simulink library "ee_lib_for_power_systems"  (R2025b)
settings: Solver=VariableStepAuto  StartTime=0.0  StopTime=10.0  RelTol=1e-3
undefined symbols (not defined in the file or in its folder): Alt_PolePair_Count, C3m, DiscIntNeverNeededParam, Enum1to12, L3m, Total_SM_Inertia, Ts
libraries: fl_lib x291, nesl_utility x219, ee_lib x139, custom_lib x12, $bdroot x10, ee_sl_lib x3, eeTransforms x2, eeGeneralControl x1, simulink x1
(part 5 of 11)

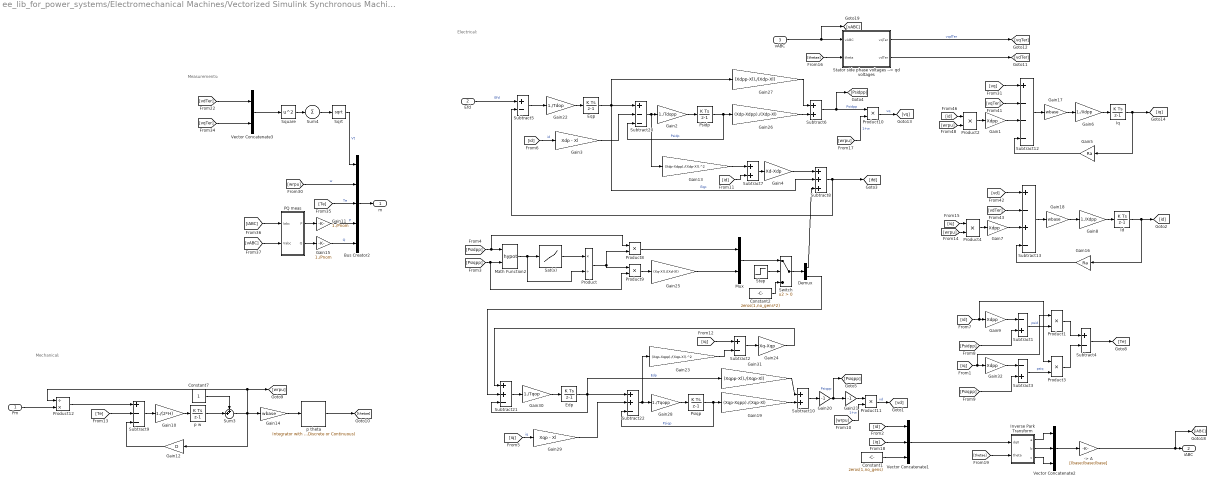
== system ee_lib_for_power_systems/Electromechanical Machines/Vectorized Simulink Synchronous Machine (PU) ==
  note: Mechanical:
  note: Electrical:
  note: Measurements:
  Pm : Inport
  Efd : Inport  Port=2
  vABC : Inport  Port=3
  -> A : Gain  Gain=[Ibase;Ibase;Ibase]
  Bus Creator2 : BusCreator  Inputs=5
  Constant1 : Constant  Value=zeros(1,no_gens)  VectorParams1D=off  SampleTime=Ts
  Constant2 : Constant  Value=zeros(1,no_gens*2)  VectorParams1D=off  SampleTime=Ts
  Constant7 : Constant  VectorParams1D=off  SampleTime=Ts
  Demux : Demux  Outputs=2
  Edp : DiscreteIntegrator  SampleTime=Ts  ICPrevOutput=DiscIntNeverNeededParam  ICPrevScaledInput=DiscIntNeverNeededParam
  Eqp : DiscreteIntegrator  SampleTime=Ts  ICPrevOutput=DiscIntNeverNeededParam  ICPrevScaledInput=DiscIntNeverNeededParam
  Gain{1,7,9,32} (4 alike) : Gain  Gain=Xdpp
  Gain10 : Gain  Gain=1./(2*H)
  Gain11 : Gain  Gain=1./Pnom
  Gain12 : Gain  Gain=D
  Gain13 : Gain  Gain=(Xdp-Xdpp)./(Xdp-Xl).^2
  Gain14 : Gain  Gain=wbase
  Gain15 : Gain  Gain=1./Pnom
  Gain16 : Gain  Gain=Ra
  Gain17 : Gain  Gain=wbase
  Gain18 : Gain  Gain=wbase
  Gain19 : Gain  Gain=(Xqp-Xqpp)./(Xqp-Xl)
  Gain2 : Gain  Gain=1./Tdopp
  Gain20 : Gain  Gain=-1
  Gain21 : Gain  Gain=-1
  Gain22 : Gain  Gain=1./Tdop
  Gain23 : Gain  Gain=(Xqp-Xqpp)./(Xqp-Xl).^2
  Gain24 : Gain  Gain=Xq-Xqp
  Gain25 : Gain  Gain=(Xq-Xl)./(Xd-Xl)
  Gain26 : Gain  Gain=(Xdp-Xdpp)./(Xdp-Xl)
  Gain27 : Gain  Gain=(Xdpp-Xl)./(Xdp-Xl)
  Gain28 : Gain  Gain=1./Tqopp
  Gain29 : Gain  Gain=Xqp - Xl
  Gain3 : Gain  Gain=Xdp - Xl
  Gain30 : Gain  Gain=1./Tqop
  Gain31 : Gain  Gain=(Xqpp-Xl)./(Xqp-Xl)
  Gain4 : Gain  Gain=Xd-Xdp
  Gain5 : Gain  Gain=Ra
  Gain6 : Gain  Gain=1./Xdpp
  Gain8 : Gain  Gain=1./Xdpp
  Id : DiscreteIntegrator  SampleTime=Ts  ICPrevOutput=DiscIntNeverNeededParam  ICPrevScaledInput=DiscIntNeverNeededParam
  Inverse Park Transform : Subsystem  mask{no_meas=no_gens}
      mask prompts: no_meas "Number of Measurements"
      settings: LibrarySourceBlock=|
      | ee_sl_lib/Mathematical
      | Transforms/Inverse
      | Park Transform
  Iq : DiscreteIntegrator  SampleTime=Ts  ICPrevOutput=DiscIntNeverNeededParam  ICPrevScaledInput=DiscIntNeverNeededParam
  Math Function2 : Math  Operator=hypot  SignedPower=on
  Mux : Mux  Inputs=2
  PQ meas : Subsystem  DialogController=POWERSYS.PowerSysDialog
      settings: LibrarySourceBlock=|
      | sps_lib/Sensors and Measurements/Power
      | (3ph, Instantaneous)
  Product : Product  Inputs=*/
  Product1 : Product
  Product{2,4,8..11} (6 alike) : Product  RndMeth=Zero
  Product12 : Product  Inputs=/*
  Product3 : Product
  Psidp : DiscreteIntegrator  SampleTime=Ts  ICPrevOutput=DiscIntNeverNeededParam  ICPrevScaledInput=DiscIntNeverNeededParam
  Psiqp : DiscreteIntegrator  SampleTime=Ts  ICPrevOutput=DiscIntNeverNeededParam  ICPrevScaledInput=DiscIntNeverNeededParam
  Sat(x) : Lookup_n-D  NumberOfTableDimensions=1  LookupTableObject=''  BreakpointsForDimension1=xVec  Table=SatX  InternalRulePriority=Speed  RndMeth=Simplest
  Sqrt : Sqrt
  Square : Math  Operator=square
  Stator side phase voltages --> qd voltages : Subsystem
  Step : Step  SampleTime=Ts
  Subtract1 : Sum  Inputs=-+
  Subtract10 : Sum
  Subtract12 : Sum  Inputs=+---
  Subtract13 : Sum  Inputs=+-+-
  Subtract2 : Sum  Inputs=+-
  Subtract20 : Sum  Inputs=+--
  Subtract21 : Sum  Inputs=++-
  Subtract22 : Sum  Inputs=++-
  Subtract3 : Sum  Inputs=-+
  Subtract4 : Sum  Inputs=+-
  Subtract5 : Sum  Inputs=+-
  Subtract6 : Sum
  Subtract7 : Sum
  Subtract8 : Sum  Inputs=+++
  Subtract9 : Sum  Inputs=+--
  Sum3 : Sum  Inputs=++|
  Sum4 : Sum  Inputs=1  CollapseMode=Specified dimension  InputSameDT=on  SaturateOnIntegerOverflow=on  OutDataTypeStr=Inherit: Same as first input
  Switch : Switch  Criteria=u2 > Threshold  SaturateOnIntegerOverflow=off  InputSameDT=off
  Vector Concatenate1 : Concatenate  NumInputs=3  Mode=Multidimensional array
  Vector Concatenate2 : Concatenate  NumInputs=3  Mode=Multidimensional array
  Vector Concatenate3 : Concatenate  Mode=Multidimensional array
  "p theta " : eeGeneralControl/Integrator with Wrapped State (Discrete or Continuous)  LabelModeActiveChoice=Discrete  WrappedStateUpperValue=2*pi  WrappedStateLowerValue=0  x0=0  Ts=Ts
      settings: LibrarySourceBlock=|
      | eeSinusoidalMeasurementV2/Sinusoidal Measurement
      | (PLL)/Angle/Integrator with
      | Wrapped State
      | (Discrete or Continuous)
  p w : DiscreteIntegrator  gainval=1  SampleTime=-1  ICPrevOutput=DiscIntNeverNeededParam  ICPrevScaledInput=DiscIntNeverNeededParam
  m : Outport
  iABC : Outport  Port=2
  wires:
  Constant2/1 -> Switch/3
  Demux/2 -> Subtract21/2
  Demux/1 -> Subtract8/3
  Switch/1 -> Demux/1
  Step/1 -> Switch/2
  Mux/1 -> Switch/1
  Gain25/1 -> Mux/2
  Product8/1 -> Mux/1
  Product/1 -> Product9/1, Product8/2
  Math Function2/1 -> Product/2, Sat(x)/1
  Subtract4/1 -> [Te]
  Product1/1 -> Subtract4/1
  Product3/1 -> Subtract4/2
  [Psiqpp] -> Subtract3/2
  Gain32/1 -> Subtract3/1
  [iq] -> Gain32/1, Product1/1
  Subtract3/1 -> Product3/2  "psiq"
  Subtract1/1 -> Product1/2  "psid"
  Sqrt/1 -> Bus Creator2/1  "Vt"
  Sat(x)/1 -> Product/1
  [thetae] -> Inverse Park Transform/2(theta)
  [thetae] -> Stator side phase voltages --> qd voltages/2(theta)
  "p theta "/1 -> [thetae]
  [vdTer] -> Subtract13/2
  Subtract13/1 -> Gain18/1
  Gain24/1 -> Subtract21/1
  Product4/1 -> Gain7/1
  Gain22/1 -> Eqp/1
  [iq] -> Product4/1
  [id] -> Gain9/1, Product3/1
  Psiqp/1 -> Subtract22/3, Gain19/1  "Psiqp"
  Gain28/1 -> Psiqp/1
  [iq] -> Gain29/1  "iq"
  Gain9/1 -> Subtract1/1
  Product9/1 -> Gain25/1
  [id] -> Subtract7/2
  Id/1 -> Gain16/1, [id]
  Gain21/1 -> Product11/1
  Gain1/1 -> Subtract12/3
  Gain2/1 -> Psidp/1
  Iq/1 -> [iq], Gain5/1
  [wrpu] -> Product4/2
  Gain8/1 -> Id/1
  [Psidpp] -> Subtract1/2
  [wrpu] -> Product11/2  "1+w"
  [vq] -> Subtract12/1
  Gain16/1 -> Subtract13/4
  [id] -> Product2/1
  Gain6/1 -> Iq/1
  [vqTer] -> Subtract12/2
  Product2/1 -> Gain1/1
  [wrpu] -> Product2/2
  Gain5/1 -> Subtract12/4
  Subtract5/1 -> Gain22/1
  Gain30/1 -> Edp/1
  Product10/1 -> [vq]  "vq"
  Product11/1 -> [vd]  "vd"
  [wrpu] -> Product10/2  "1+w"
  Gain17/1 -> Gain6/1
  [vd] -> Subtract13/1
  Gain31/1 -> Subtract10/1
  Gain18/1 -> Gain8/1
  Subtract21/1 -> Gain30/1
  Gain4/1 -> Subtract8/1
  Subtract7/1 -> Gain4/1
  Efd/1 -> Subtract5/1  "Efd"
  Gain3/1 -> Subtract20/2
  Eqp/1 -> Gain27/1, Subtract20/1, Subtract8/2  "Eqp"
  Subtract8/1 -> Subtract5/2, [ifd]
  Subtract2/1 -> Gain24/1
  Gain29/1 -> Subtract22/2
  [Psidpp] -> Math Function2/1, Product8/1
  [id] -> Gain3/1  "id"
  [Psiqpp] -> Math Function2/2, Product9/2
  Subtract22/1 -> Gain28/1, Gain23/1
  Subtract6/1 -> Product10/1, [Psidpp]  "Psidpp"
  Subtract20/1 -> Gain2/1, Gain13/1
  Gain19/1 -> Subtract10/2
  Gain13/1 -> Subtract7/1
  Gain26/1 -> Subtract6/2
  Gain7/1 -> Subtract13/3
  Gain27/1 -> Subtract6/1
  Subtract12/1 -> Gain17/1
  Gain20/1 -> Gain21/1, [Psiqpp]  "Psiqpp"
  Subtract10/1 -> Gain20/1
  [iq] -> Subtract2/1
  Psidp/1 -> Gain26/1, Subtract20/3  "Psidp"
  Gain23/1 -> Subtract2/2
  Edp/1 -> Subtract21/3, Subtract22/1, Gain31/1  "Edp"
  [Te] -> Subtract9/2
  Vector Concatenate1/1 -> Inverse Park Transform/1(dq0)
  -> A/1 -> iABC/1, [iABC]
  vABC/1 -> Stator side phase voltages --> qd voltages/1(vABC), [vABC]
  Stator side phase voltages --> qd voltages/2(vdTer) -> [vdTer]
  Gain15/1 -> Bus Creator2/5  "Q"
  Gain11/1 -> Bus Creator2/4  "P"
  PQ meas/1(Q) -> Gain15/1
  PQ meas/2(P) -> Gain11/1
  [vABC] -> PQ meas/1(Vabc)
  [iABC] -> PQ meas/2(Iabc)
  [Te] -> Bus Creator2/3  "Te"
  [wrpu] -> Bus Creator2/2  "w"
  [vqTer] -> Vector Concatenate3/2
  Vector Concatenate2/1 -> -> A/1
  Inverse Park Transform/3(c) -> Vector Concatenate2/3
  Inverse Park Transform/1(a) -> Vector Concatenate2/1
  Inverse Park Transform/2(b) -> Vector Concatenate2/2
  Constant7/1 -> Sum3/1
  Stator side phase voltages --> qd voltages/1(vqTer) -> [vqTer]  "vqdTer"
  Sum3/1 -> Gain12/1, Gain14/1, [wrpu], Product12/1
  Pm/1 -> Product12/2
  p w/1 -> Sum3/2
  Subtract9/1 -> Gain10/1
  Gain10/1 -> p w/1
  Product12/1 -> Subtract9/1
  Gain12/1 -> Subtract9/3
  Gain14/1 -> "p theta "/1
  [id] -> Vector Concatenate1/1
  [iq] -> Vector Concatenate1/2
  Bus Creator2/1 -> m/1
  [vdTer] -> Vector Concatenate3/1
  Constant1/1 -> Vector Concatenate1/3
  Vector Concatenate3/1 -> Square/1
  Square/1 -> Sum4/1
  Sum4/1 -> Sqrt/1

== system ee_lib_for_power_systems/Network Components/ Three-Winding Transformer PU (Three-Phase) ==
  Transformer : ee_lib/Passive/Transformers/Three-Winding Transformer (Three-Phase)  SRated=Pnom_Fnom(1) A*V  FRated=Pnom_Fnom(2) Hz  Winding1Connection=ee.enum.windingconnection.Yg  VRated1=V1 V  Winding2Connection=ee.enum.windingconnection.delta1  VRated2=V2 V  Winding3Connection=ee.enum.windingconnection.Y  VRated3=V3 V  CoreType=ee.enum.coretype.fivelimb  pu_Rw1=R1_pu  pu_Rw2=R2_pu  pu_Rw3=R3_pu  leakage_reactance_option=ee.enum.transformer_leakage.include  pu_Xl1=L1_pu  pu_Xl2=L2_pu  pu_Xl3=L3_pu  magnetizing_resistance_option=ee.enum.transformer_magnetizingResistance.include  pu_Rm=Rm_pu  magnetizing_reactance_option=ee.enum.transformer_magnetizingReactance.include  saturation_option=ee.enum.transformer_saturation.exclude  pu_Xm=Lm_pu  current_data=[0, .002, 1, 2]  magnetic_flux_data=[0, 1.2, 1.5, 1.51]  pu_X0=0.5  i1_specify=off  i1_priority=None  i1=[0, 0, 0] A  i1_nominal_specify=off  i1_nominal_value=1  i1_nominal_unit=A  i2_specify=off  i2_priority=None  i2=[0, 0, 0] A  i2_nominal_specify=off  i2_nominal_value=1  i2_nominal_unit=A  i3_specify=off  i3_priority=None  i3=[0, 0, 0] A  i3_nominal_specify=off  i3_nominal_value=1  i3_nominal_unit=A  phi_specify=off  phi_priority=None  phi=[0, 0, 0]  phi_nominal_specify=off  phi_nominal_value=1  phi_nominal_unit=1  power_dissipated_specify=off  power_dissipated_priority=None  power_dissipated=0 W  power_dissipated_nominal_specify=off  power_dissipated_nominal_value=1  power_dissipated_nominal_unit=W
      settings: InternalSimscapePortConfiguration="{\"Bottom\":[],\"Left\":[{\"id\":\"N1\",\"label\":\"~1\",\"type\":\"foundation.electrical.three_phase\"}],\"Right\":[{\"id\":\"N2\",\"label\":\"~2\",\"type\":\"foundation.electrical.three_phase\"},{\"id\":\"N3\",\"label\":\"~3\",\"type\":\"foundation.electrical.three_phase\"}],\"Top\":[]}"
  ~1 : PMIOPort  Side=Left
  ~2 : PMIOPort  Port=2  Side=Right
  ~3 : PMIOPort  Port=3  Side=Right
  connections:
  Transformer/LConn1 -- ~1/RConn1
  ~2/RConn1 -- Transformer/RConn1
  ~3/RConn1 -- Transformer/RConn2

== system ee_lib_for_power_systems/Network Components/3-Phase Branch Positive Sequence PU ==
  C1 : ee_lib/Passive/RLC Assemblies/RLC (Three-Phase)  port_option=ee.enum.threePhasePort.composite  component_structure=ee.enum.rlc.structure.C  R=1 Ohm  L=1e-3 H  C=B/(Vnom2^2/Pnom_Fnom(1))/(2*pi*Pnom_Fnom(2))/2 F  R_parasitic=0 Ohm  G_parasitic=0 1/Ohm  IL_specify=off  IL_priority=High  IL=[0, 0, 0] A  IL_nominal_specify=off  IL_nominal_value=1  IL_nominal_unit=A  VC_specify=on  VC_priority=Low  VC=[0, 0, 0] V  VC_nominal_specify=off  VC_nominal_value=1  VC_nominal_unit=V
      settings: InternalSimscapePortConfiguration="{\"Bottom\":[],\"Left\":[{\"id\":\"N1\",\"label\":\"~1\",\"type\":\"foundation.electrical.three_phase\"}],\"Right\":[{\"id\":\"N2\",\"label\":\"~2\",\"type\":\"foundation.electrical.three_phase\"}],\"Top\":[]}"
  C2 : ee_lib/Passive/RLC Assemblies/RLC (Three-Phase)  port_option=ee.enum.threePhasePort.composite  component_structure=ee.enum.rlc.structure.C  R=1 Ohm  L=1e-3 H  C=B/(Vnom2^2/Pnom_Fnom(1))/(2*pi*Pnom_Fnom(2))/2 F  R_parasitic=0 Ohm  G_parasitic=0 1/Ohm  IL_specify=off  IL_priority=High  IL=[0, 0, 0] A  IL_nominal_specify=off  IL_nominal_value=1  IL_nominal_unit=A  VC_specify=on  VC_priority=Low  VC=[0, 0, 0] V  VC_nominal_specify=off  VC_nominal_value=1  VC_nominal_unit=V
      settings: InternalSimscapePortConfiguration="{\"Bottom\":[],\"Left\":[{\"id\":\"N1\",\"label\":\"~1\",\"type\":\"foundation.electrical.three_phase\"}],\"Right\":[{\"id\":\"N2\",\"label\":\"~2\",\"type\":\"foundation.electrical.three_phase\"}],\"Top\":[]}"
  RLC : ee_lib/Passive/RLC Assemblies/RLC (Three-Phase)  port_option=ee.enum.threePhasePort.composite  component_structure=ee.enum.rlc.structure.SeriesRL  R=R*Vnom2^2/Pnom_Fnom(1) Ohm  L=X*Vnom2^2/Pnom_Fnom(1)/(2*pi*Pnom_Fnom(2)) H  C=-1/(Vnom2^2/Pnom_Fnom(1))/(2*pi*Pnom_Fnom(2))/X F  R_parasitic=0 Ohm  G_parasitic=0 1/Ohm  IL_specify=on  IL_priority=Low  IL=[0, 0, 0] A  IL_nominal_specify=off  IL_nominal_value=1  IL_nominal_unit=A  VC_specify=on  VC_priority=Low  VC=[0, 0, 0] V  VC_nominal_specify=off  VC_nominal_value=1  VC_nominal_unit=V
      settings: InternalSimscapePortConfiguration="{\"Bottom\":[],\"Left\":[{\"id\":\"N1\",\"label\":\"~1\",\"type\":\"foundation.electrical.three_phase\"}],\"Right\":[{\"id\":\"N2\",\"label\":\"~2\",\"type\":\"foundation.electrical.three_phase\"}],\"Top\":[]}"
  Tx : Subsystem  Commented=through  mask{wr=Vnom1/Vnom2}
      mask prompts: wr "Winding Ratio (V1/V2)"
  gnd1 : ee_lib/Connectors & References/Grounded Neutral (Three-Phase)
  gnd2 : ee_lib/Connectors & References/Grounded Neutral (Three-Phase)
  ~1 : PMIOPort  Side=Left
  ~2 : PMIOPort  Port=2  Side=Right
  connections:
  Tx/RConn1 -- RLC/LConn1 -- C1/LConn1
  Tx/LConn1 -- ~1/RConn1
  RLC/RConn1 -- ~2/RConn1 -- C2/LConn1
  gnd1/LConn1 -- C1/RConn1
  gnd2/LConn1 -- C2/RConn1

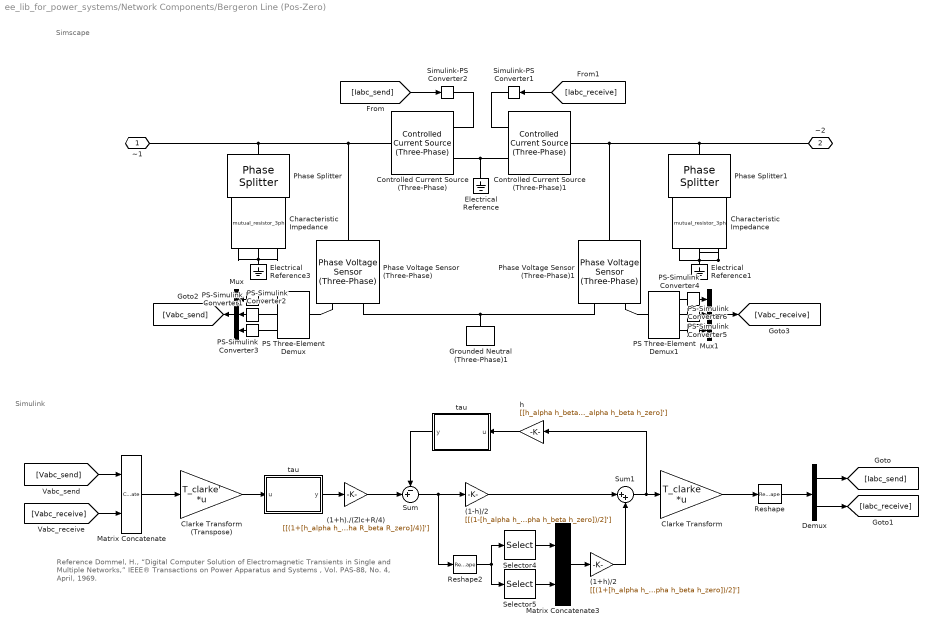
== system ee_lib_for_power_systems/Network Components/Bergeron Line (Pos-Zero) ==
  note: Simulink
  note: Simscape
  note: Reference
      |
      | Dommel, H., “Digital Computer Solution of Electromagnetic Transients in Single and Multiple Networks,” IEEE® Transactions on Power Apparatus and Systems , Vol. PAS-88, No. 4, April, 1969.
  (1+h)./(Zlc+R/4) : Gain  Gain=[[(1+[h_alpha h_beta h_zero])./([Zlc_alpha Zlc_beta Zlc_zero]+[R_alpha R_beta R_zero]/4)]' [(1+[h_alpha h_beta h_zero])./([Zlc_alpha Zlc_beta Zlc_zero]+[R_alpha R_beta R_zero]/4)]']
  (1+h)/2 : Gain  Gain=[[(1+[h_alpha h_beta h_zero])/2]' [(1+[h_alpha h_beta h_zero])/2]']
  (1-h)/2 : Gain  Gain=[[(1-[h_alpha h_beta h_zero])/2]' [(1-[h_alpha h_beta h_zero])/2]']
  Characteristic Impedance : custom_lib/mutual_resistor_3ph  R=Zc Ohm  V_specify=off  V_priority=None  V=zeros(3, 1) V  V_nominal_specify=off  V_nominal_value=1  V_nominal_unit=V  I_specify=off  I_priority=High  I=zeros(3, 1) A  I_nominal_specify=off  I_nominal_value=1  I_nominal_unit=A
      settings: InternalSimscapePortConfiguration="{\"Bottom\":[],\"Left\":[{\"id\":\"p1\",\"label\":\"p1\",\"type\":\"foundation.electrical.electrical\"},{\"id\":\"p2\",\"label\":\"p2\",\"type\":\"foundation.electrical.electrical\"},{\"id\":\"p3\",\"label\":\"p3\",\"type\":\"foundation.electrical.electrical\"}],\"Right\":[{\"id\":\"n1\",\"label\":\"n1\",\"type\":\"foundation.electrical.electrical\"},{\"id\":\"n2\",\"label\":\"n2\",\"type\":\"foundation.electrical.electrical\"},{\"id\":\"n3\",\"label\":\"n3\",\"type\":\"foundation.electrical.electrical\"}],\"Top\":[]}"
  "Characteristic Impedance " : custom_lib/mutual_resistor_3ph  R=Zc Ohm  V_specify=off  V_priority=None  V=zeros(3, 1) V  V_nominal_specify=off  V_nominal_value=1  V_nominal_unit=V  I_specify=off  I_priority=High  I=zeros(3, 1) A  I_nominal_specify=off  I_nominal_value=1  I_nominal_unit=A
      settings: InternalSimscapePortConfiguration="{\"Bottom\":[],\"Left\":[{\"id\":\"p1\",\"label\":\"p1\",\"type\":\"foundation.electrical.electrical\"},{\"id\":\"p2\",\"label\":\"p2\",\"type\":\"foundation.electrical.electrical\"},{\"id\":\"p3\",\"label\":\"p3\",\"type\":\"foundation.electrical.electrical\"}],\"Right\":[{\"id\":\"n1\",\"label\":\"n1\",\"type\":\"foundation.electrical.electrical\"},{\"id\":\"n2\",\"label\":\"n2\",\"type\":\"foundation.electrical.electrical\"},{\"id\":\"n3\",\"label\":\"n3\",\"type\":\"foundation.electrical.electrical\"}],\"Top\":[]}"
  Clarke Transform (Transpose) : Gain  Gain=T_clarke'  Multiplication=Matrix(K*u)
  "Clarke Transform " : Gain  Gain=T_clarke  Multiplication=Matrix(K*u)
  Controlled Current Source (Three-Phase) : ee_lib/Sources/Controlled Current Source (Three-Phase)  input_option=ee.enum.inputType.instantaneous  FRated=60 Hz
      settings: InternalSimscapePortConfiguration="{\"Bottom\":[],\"Left\":[{\"id\":\"S\",\"label\":\"S\",\"type\":\"input\"},{\"id\":\"n\",\"label\":\"n\",\"type\":\"foundation.electrical.electrical\"}],\"Right\":[{\"id\":\"N\",\"label\":\"~\",\"type\":\"foundation.electrical.three_phase\"}],\"Top\":[]}"
  Controlled Current Source (Three-Phase)1 : ee_lib/Sources/Controlled Current Source (Three-Phase)  input_option=ee.enum.inputType.instantaneous  FRated=60 Hz
      settings: InternalSimscapePortConfiguration="{\"Bottom\":[],\"Left\":[{\"id\":\"S\",\"label\":\"S\",\"type\":\"input\"},{\"id\":\"n\",\"label\":\"n\",\"type\":\"foundation.electrical.electrical\"}],\"Right\":[{\"id\":\"N\",\"label\":\"~\",\"type\":\"foundation.electrical.three_phase\"}],\"Top\":[]}"
  Demux : Demux  Outputs=2
  Electrical Reference : fl_lib/Electrical/Electrical Elements/Electrical Reference
  Electrical Reference1 : fl_lib/Electrical/Electrical Elements/Electrical Reference
  Electrical Reference3 : fl_lib/Electrical/Electrical Elements/Electrical Reference
  Grounded Neutral (Three-Phase)1 : ee_lib/Connectors & References/Grounded Neutral (Three-Phase)
  Matrix Concatenate : Concatenate  Mode=Multidimensional array  ConcatenateDimension=2
  Matrix Concatenate3 : Concatenate  Mode=Multidimensional array  ConcatenateDimension=2
  Mux : Mux  Inputs=3
  Mux1 : Mux  Inputs=3
  PS Three-Element Demux : fl_lib/Physical Signals/Nonlinear Operators/PS Three-Element Demux
      settings: InternalSimscapePortConfiguration="{\"Bottom\":[],\"Left\":[{\"id\":\"abc\",\"label\":\"abc\",\"type\":\"input\"}],\"Right\":[{\"id\":\"a\",\"label\":\"a\",\"type\":\"output\"},{\"id\":\"b\",\"label\":\"b\",\"type\":\"output\"},{\"id\":\"c\",\"label\":\"c\",\"type\":\"output\"}],\"Top\":[]}"
  PS Three-Element Demux1 : fl_lib/Physical Signals/Nonlinear Operators/PS Three-Element Demux
      settings: InternalSimscapePortConfiguration="{\"Bottom\":[],\"Left\":[{\"id\":\"abc\",\"label\":\"abc\",\"type\":\"input\"}],\"Right\":[{\"id\":\"a\",\"label\":\"a\",\"type\":\"output\"},{\"id\":\"b\",\"label\":\"b\",\"type\":\"output\"},{\"id\":\"c\",\"label\":\"c\",\"type\":\"output\"}],\"Top\":[]}"
  PS-Simulink Converter{1..6} (6 alike) : nesl_utility/PS-Simulink Converter  PhysicalDomain=network_engine_domain  SubClassName=ps_output  LeftPortType=input  RightPortType=output  PseudoPeriodic=off  Frequencies=[]  VectorFormat=inherit  Unit=inherit  AffineConversion=off
  Phase Splitter : ee_lib/Connectors & References/Phase Splitter
  Phase Splitter1 : ee_lib/Connectors & References/Phase Splitter
  Phase Voltage Sensor (Three-Phase) : ee_lib/Sensors & Transducers/Phase Voltage Sensor (Three-Phase)
  Phase Voltage Sensor (Three-Phase)1 : ee_lib/Sensors & Transducers/Phase Voltage Sensor (Three-Phase)
  Reshape : Reshape
  Reshape2 : Reshape
  Selector4 : Selector  InputPortWidth=6  IndexOptions=Index vector (dialog)  Indices=4:6  OutputSizes=1
  Selector5 : Selector  InputPortWidth=6  IndexOptions=Index vector (dialog)  Indices=1:3  OutputSizes=1
  Simulink-PS Converter1 : nesl_utility/Simulink-PS Converter  PhysicalDomain=network_engine_domain  SubClassName=ps_input  LeftPortType=input  RightPortType=output  PseudoPeriodic=off  Frequencies=[]  NoiseDistribution=none  NoiseParameters=[]  Unit=1  AffineConversion=off  FilteringAndDerivatives=provide  SimscapeFilterOrder=2  InputFilterTimeConstant=0.001  UdotUserProvided=0
  Simulink-PS Converter2 : nesl_utility/Simulink-PS Converter  PhysicalDomain=network_engine_domain  SubClassName=ps_input  LeftPortType=input  RightPortType=output  PseudoPeriodic=off  Frequencies=[]  NoiseDistribution=none  NoiseParameters=[]  Unit=1  AffineConversion=off  FilteringAndDerivatives=provide  SimscapeFilterOrder=2  InputFilterTimeConstant=0.001  UdotUserProvided=0
  Sum : Sum  Inputs=-+|
  Sum1 : Sum  Inputs=|++
  h : Gain  Gain=[[h_alpha h_beta h_zero]' [h_alpha h_beta h_zero]']
  tau : Subsystem  mask{tau=[tau_alpha tau_beta tau_zero], Ts=Ts}
      mask prompts: tau "Transport Delay (s)"; Ts "Sample Time (s)"
      mask DialogControl DescGroupVar Prompt: Discrete Transport Delay
      mask DialogControl DescTextVar Prompt: This block implements a discrete transport delay. When the transport delay is not an integer multiple of the sample time, the remainder is averaged.
      mask DialogControl param Prompt: Parameters
      mask Display: disp('Discrete\nTransport\nDelay')
  "tau " : Subsystem  mask{tau=[tau_alpha tau_beta tau_zero], Ts=Ts}
      mask prompts: tau "Transport Delay (s)"; Ts "Sample Time (s)"
      mask DialogControl DescGroupVar Prompt: Discrete Transport Delay
      mask DialogControl DescTextVar Prompt: This block implements a discrete transport delay. When the transport delay is not an integer multiple of the sample time, the remainder is averaged.
      mask DialogControl param Prompt: Parameters
      mask Display: disp('Discrete\nTransport\nDelay')
  ~1 : PMIOPort  Side=Left
  ~2 : PMIOPort  Port=2  Side=Right
  wires:
  Selector5/1 -> Matrix Concatenate3/2
  Matrix Concatenate/1 -> Clarke Transform (Transpose)/1
  (1-h)/2/1 -> Sum1/1
  (1+h)./(Zlc+R/4)/1 -> Sum/2
  Demux/2 -> [Iabc_receive]
  Demux/1 -> [Iabc_send]
  Selector4/1 -> Matrix Concatenate3/1
  "Clarke Transform "/1 -> Reshape/1
  Reshape/1 -> Demux/1
  "tau "/1(y) -> Sum/1
  [Vabc_send] -> Matrix Concatenate/1
  (1+h)/2/1 -> Sum1/2
  Matrix Concatenate3/1 -> (1+h)/2/1
  h/1 -> "tau "/1(u)
  tau/1(y) -> (1+h)./(Zlc+R/4)/1
  Reshape2/1 -> Selector4/1, Selector5/1
  Sum1/1 -> "Clarke Transform "/1, h/1
  [Vabc_receive] -> Matrix Concatenate/2
  Sum/1 -> (1-h)/2/1, Reshape2/1
  Clarke Transform (Transpose)/1 -> tau/1(u)
  Mux/1 -> [Vabc_send]
  Mux1/1 -> [Vabc_receive]
  PS-Simulink Converter2/1 -> Mux/2
  PS-Simulink Converter1/1 -> Mux/1
  PS-Simulink Converter3/1 -> Mux/3
  PS-Simulink Converter5/1 -> Mux1/2
  PS-Simulink Converter6/1 -> Mux1/3
  PS-Simulink Converter4/1 -> Mux1/1
  [Iabc_send] -> Simulink-PS Converter2/1
  [Iabc_receive] -> Simulink-PS Converter1/1
  connections:
  "Characteristic Impedance "/LConn1 -- Phase Splitter1/RConn1
  "Characteristic Impedance "/LConn2 -- Phase Splitter1/RConn2
  "Characteristic Impedance "/LConn3 -- Phase Splitter1/RConn3
  Electrical Reference1/LConn1 -- "Characteristic Impedance "/RConn2 -- "Characteristic Impedance "/RConn1 -- "Characteristic Impedance "/RConn3
  Characteristic Impedance/LConn3 -- Phase Splitter/RConn3
  Characteristic Impedance/LConn2 -- Phase Splitter/RConn2
  Characteristic Impedance/LConn1 -- Phase Splitter/RConn1
  Characteristic Impedance/RConn2 -- Characteristic Impedance/RConn3 -- Characteristic Impedance/RConn1 -- Electrical Reference3/LConn1
  PS Three-Element Demux1/LConn1 -- Phase Voltage Sensor (Three-Phase)1/RConn1
  ~1/RConn1 -- Phase Splitter/LConn1 -- Phase Voltage Sensor (Three-Phase)/LConn1 -- Controlled Current Source (Three-Phase)/RConn1
  PS-Simulink Converter6/LConn1 -- PS Three-Element Demux1/RConn3
  PS-Simulink Converter3/LConn1 -- PS Three-Element Demux/RConn3
  PS-Simulink Converter2/LConn1 -- PS Three-Element Demux/RConn2
  PS-Simulink Converter5/LConn1 -- PS Three-Element Demux1/RConn2
  PS-Simulink Converter4/LConn1 -- PS Three-Element Demux1/RConn1
  PS Three-Element Demux/LConn1 -- Phase Voltage Sensor (Three-Phase)/RConn1
  Controlled Current Source (Three-Phase)1/RConn1 -- Phase Splitter1/LConn1 -- ~2/RConn1 -- Phase Voltage Sensor (Three-Phase)1/LConn1
  Grounded Neutral (Three-Phase)1/LConn1 -- Phase Voltage Sensor (Three-Phase)/RConn2 -- Phase Voltage Sensor (Three-Phase)1/RConn2
  PS-Simulink Converter1/LConn1 -- PS Three-Element Demux/RConn1
  Simulink-PS Converter2/RConn1 -- Controlled Current Source (Three-Phase)/LConn1
  Controlled Current Source (Three-Phase)/LConn2 -- Controlled Current Source (Three-Phase)1/LConn2 -- Electrical Reference/LConn1
  Controlled Current Source (Three-Phase)1/LConn1 -- Simulink-PS Converter1/RConn1

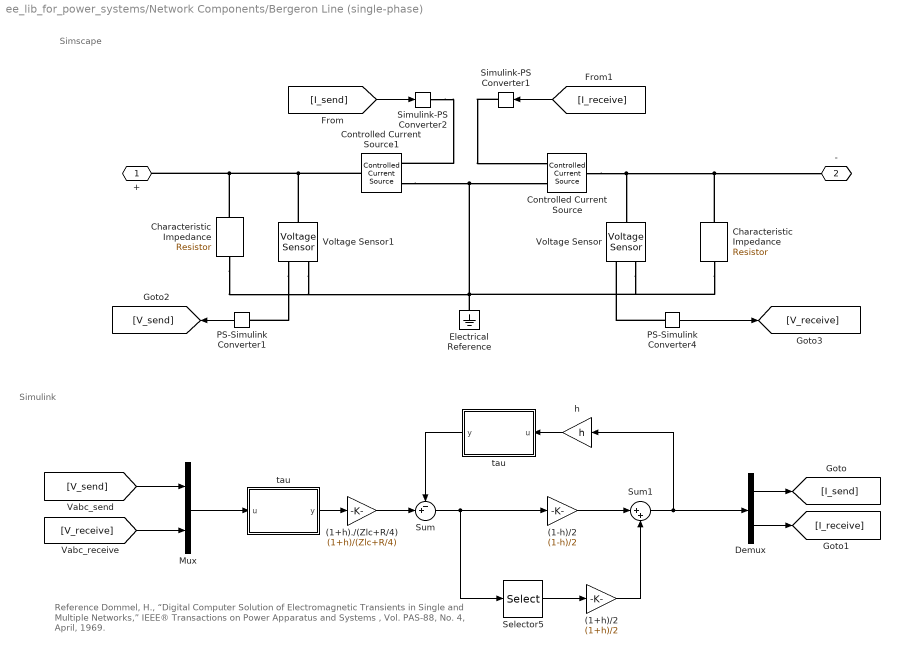
== system ee_lib_for_power_systems/Network Components/Bergeron Line (single-phase) ==
  note: Reference
      |
      | Dommel, H., “Digital Computer Solution of Electromagnetic Transients in Single and Multiple Networks,” IEEE® Transactions on Power Apparatus and Systems , Vol. PAS-88, No. 4, April, 1969.
  note: Simscape
  note: Simulink
  (1+h)./(Zlc+R/4) : Gain  Gain=(1+h)/(Zlc+R/4)
  (1+h)/2 : Gain  Gain=(1+h)/2
  (1-h)/2 : Gain  Gain=(1-h)/2
  Characteristic Impedance : fl_lib/Electrical/Electrical Elements/Resistor  R=Zc Ohm  i_specify=off  i_priority=None  i=0 A  i_nominal_specify=off  i_nominal_value=1  i_nominal_unit=A  v_specify=off  v_priority=None  v=0 V  v_nominal_specify=off  v_nominal_value=1  v_nominal_unit=V
  "Characteristic Impedance " : fl_lib/Electrical/Electrical Elements/Resistor  R=Zc Ohm  i_specify=off  i_priority=None  i=0 A  i_nominal_specify=off  i_nominal_value=1  i_nominal_unit=A  v_specify=off  v_priority=None  v=0 V  v_nominal_specify=off  v_nominal_value=1  v_nominal_unit=V
  Controlled Current Source : fl_lib/Electrical/Electrical Sources/Controlled Current Source
  Controlled Current Source1 : fl_lib/Electrical/Electrical Sources/Controlled Current Source
  Demux : Demux  Outputs=2
  Electrical Reference : fl_lib/Electrical/Electrical Elements/Electrical Reference
  Mux : Mux  Inputs=2
  PS-Simulink Converter1 : nesl_utility/PS-Simulink Converter  PhysicalDomain=network_engine_domain  SubClassName=ps_output  LeftPortType=input  RightPortType=output  PseudoPeriodic=off  Frequencies=[]  VectorFormat=inherit  Unit=inherit  AffineConversion=off
  PS-Simulink Converter4 : nesl_utility/PS-Simulink Converter  PhysicalDomain=network_engine_domain  SubClassName=ps_output  LeftPortType=input  RightPortType=output  PseudoPeriodic=off  Frequencies=[]  VectorFormat=inherit  Unit=inherit  AffineConversion=off
  Selector5 : Selector  InputPortWidth=2  IndexOptions=Index vector (dialog)  Indices=[2 1]  OutputSizes=1
  Simulink-PS Converter1 : nesl_utility/Simulink-PS Converter  PhysicalDomain=network_engine_domain  SubClassName=ps_input  LeftPortType=input  RightPortType=output  PseudoPeriodic=off  Frequencies=[]  NoiseDistribution=none  NoiseParameters=[]  Unit=1  AffineConversion=off  FilteringAndDerivatives=provide  SimscapeFilterOrder=2  InputFilterTimeConstant=0.001  UdotUserProvided=0
  Simulink-PS Converter2 : nesl_utility/Simulink-PS Converter  PhysicalDomain=network_engine_domain  SubClassName=ps_input  LeftPortType=input  RightPortType=output  PseudoPeriodic=off  Frequencies=[]  NoiseDistribution=none  NoiseParameters=[]  Unit=1  AffineConversion=off  FilteringAndDerivatives=provide  SimscapeFilterOrder=2  InputFilterTimeConstant=0.001  UdotUserProvided=0
  Sum : Sum  Inputs=-+|
  Sum1 : Sum  Inputs=|++
  Voltage Sensor : fl_lib/Electrical/Electrical Sensors/Voltage Sensor
  Voltage Sensor1 : fl_lib/Electrical/Electrical Sensors/Voltage Sensor
  h : Gain  Gain=h
  tau : Subsystem  mask{tau=tau, Ts=Ts}
      mask prompts: tau "Transport Delay (s)"; Ts "Sample Time (s)"
      mask DialogControl DescGroupVar Prompt: Discrete Transport Delay
      mask DialogControl DescTextVar Prompt: This block implements a discrete transport delay. When the transport delay is not an integer multiple of the sample time, the remainder is averaged.
      mask DialogControl param Prompt: Parameters
      mask Display: disp('Discrete\nTransport\nDelay')
  "tau " : Subsystem  mask{tau=tau, Ts=Ts}
      mask prompts: tau "Transport Delay (s)"; Ts "Sample Time (s)"
      mask DialogControl DescGroupVar Prompt: Discrete Transport Delay
      mask DialogControl DescTextVar Prompt: This block implements a discrete transport delay. When the transport delay is not an integer multiple of the sample time, the remainder is averaged.
      mask DialogControl param Prompt: Parameters
      mask Display: disp('Discrete\nTransport\nDelay')
  + : PMIOPort  Side=Left
  - : PMIOPort  Port=2  Side=Right
  wires:
  [I_receive] -> Simulink-PS Converter1/1
  [I_send] -> Simulink-PS Converter2/1
  PS-Simulink Converter4/1 -> [V_receive]
  PS-Simulink Converter1/1 -> [V_send]
  Mux/1 -> tau/1(u)
  [V_receive] -> Mux/2
  Sum/1 -> Selector5/1, (1-h)/2/1
  tau/1(y) -> (1+h)./(Zlc+R/4)/1
  h/1 -> "tau "/1(u)
  Selector5/1 -> (1+h)/2/1
  (1+h)/2/1 -> Sum1/2
  [V_send] -> Mux/1
  "tau "/1(y) -> Sum/1
  Sum1/1 -> Demux/1, h/1
  Demux/1 -> [I_send]
  Demux/2 -> [I_receive]
  (1+h)./(Zlc+R/4)/1 -> Sum/2
  (1-h)/2/1 -> Sum1/1
  connections:
  Simulink-PS Converter1/RConn1 -- Controlled Current Source/RConn1
  Electrical Reference/LConn1 -- "Characteristic Impedance "/RConn1 -- Voltage Sensor1/RConn2 -- Characteristic Impedance/RConn1 -- Voltage Sensor/RConn2 -- Controlled Current Source1/RConn2 -- Controlled Current Source/RConn2
  Simulink-PS Converter2/RConn1 -- Controlled Current Source1/RConn1
  Voltage Sensor1/RConn1 -- PS-Simulink Converter1/LConn1
  Controlled Current Source1/LConn1 -- Voltage Sensor1/LConn1 -- "Characteristic Impedance "/LConn1 -- +/RConn1
  PS-Simulink Converter4/LConn1 -- Voltage Sensor/RConn1
  Controlled Current Source/LConn1 -- -/RConn1 -- Characteristic Impedance/LConn1 -- Voltage Sensor/LConn1

== system ee_lib_for_power_systems/Network Components/Connector Bergeron Line (Pos-Zero) ==
  note: Simulink
  note: Simscape
  note: Reference
      |
      | Dommel, H., “Digital Computer Solution of Electromagnetic Transients in Single and Multiple Networks,” IEEE® Transactions on Power Apparatus and Systems , Vol. PAS-88, No. 4, April, 1969.
  receive : Inport
  (1+h)./(Zlc+R/4) : Gain  Gain=[(1+[h_alpha h_beta h_zero])./([Zlc_alpha Zlc_beta Zlc_zero]+[R_alpha R_beta R_zero]/4)]'
  (1+h)/2 : Gain  Gain=[(1+[h_alpha h_beta h_zero])/2]'
  (1-h)/2 : Gain  Gain=[(1-[h_alpha h_beta h_zero])/2]'
  Characteristic Impedance : custom_lib/mutual_resistor_3ph  R=Zc Ohm  V_specify=off  V_priority=None  V=zeros(3, 1) V  V_nominal_specify=off  V_nominal_value=1  V_nominal_unit=V  I_specify=off  I_priority=High  I=zeros(3, 1) A  I_nominal_specify=off  I_nominal_value=1  I_nominal_unit=A
      settings: InternalSimscapePortConfiguration="{\"Bottom\":[],\"Left\":[{\"id\":\"p1\",\"label\":\"p1\",\"type\":\"foundation.electrical.electrical\"},{\"id\":\"p2\",\"label\":\"p2\",\"type\":\"foundation.electrical.electrical\"},{\"id\":\"p3\",\"label\":\"p3\",\"type\":\"foundation.electrical.electrical\"}],\"Right\":[{\"id\":\"n1\",\"label\":\"n1\",\"type\":\"foundation.electrical.electrical\"},{\"id\":\"n2\",\"label\":\"n2\",\"type\":\"foundation.electrical.electrical\"},{\"id\":\"n3\",\"label\":\"n3\",\"type\":\"foundation.electrical.electrical\"}],\"Top\":[]}"
  Clarke Transform (Transpose) : Gain  Gain=T_clarke'  Multiplication=Matrix(K*u)
  "Clarke Transform " : Gain  Gain=T_clarke  Multiplication=Matrix(K*u)
  Controlled Current Source (Three-Phase) : ee_lib/Sources/Controlled Current Source (Three-Phase)  input_option=ee.enum.inputType.instantaneous  FRated=60 Hz
      settings: InternalSimscapePortConfiguration="{\"Bottom\":[],\"Left\":[{\"id\":\"S\",\"label\":\"S\",\"type\":\"input\"},{\"id\":\"n\",\"label\":\"n\",\"type\":\"foundation.electrical.electrical\"}],\"Right\":[{\"id\":\"N\",\"label\":\"~\",\"type\":\"foundation.electrical.three_phase\"}],\"Top\":[]}"
  Electrical Reference : fl_lib/Electrical/Electrical Elements/Electrical Reference
  Electrical Reference3 : fl_lib/Electrical/Electrical Elements/Electrical Reference
  Grounded Neutral (Three-Phase)1 : ee_lib/Connectors & References/Grounded Neutral (Three-Phase)
  Mux : Mux  Inputs=3
  PS Three-Element Demux : fl_lib/Physical Signals/Nonlinear Operators/PS Three-Element Demux
      settings: InternalSimscapePortConfiguration="{\"Bottom\":[],\"Left\":[{\"id\":\"abc\",\"label\":\"abc\",\"type\":\"input\"}],\"Right\":[{\"id\":\"a\",\"label\":\"a\",\"type\":\"output\"},{\"id\":\"b\",\"label\":\"b\",\"type\":\"output\"},{\"id\":\"c\",\"label\":\"c\",\"type\":\"output\"}],\"Top\":[]}"
  PS-Simulink Converter1 : nesl_utility/PS-Simulink Converter  PhysicalDomain=network_engine_domain  SubClassName=ps_output  LeftPortType=input  RightPortType=output  PseudoPeriodic=off  Frequencies=[]  VectorFormat=inherit  Unit=inherit  AffineConversion=off
  PS-Simulink Converter2 : nesl_utility/PS-Simulink Converter  PhysicalDomain=network_engine_domain  SubClassName=ps_output  LeftPortType=input  RightPortType=output  PseudoPeriodic=off  Frequencies=[]  VectorFormat=inherit  Unit=inherit  AffineConversion=off
  PS-Simulink Converter3 : nesl_utility/PS-Simulink Converter  PhysicalDomain=network_engine_domain  SubClassName=ps_output  LeftPortType=input  RightPortType=output  PseudoPeriodic=off  Frequencies=[]  VectorFormat=inherit  Unit=inherit  AffineConversion=off
  Phase Splitter : ee_lib/Connectors & References/Phase Splitter
  Phase Voltage Sensor (Three-Phase) : ee_lib/Sensors & Transducers/Phase Voltage Sensor (Three-Phase)
  Simulink-PS Converter2 : nesl_utility/Simulink-PS Converter  PhysicalDomain=network_engine_domain  SubClassName=ps_input  LeftPortType=input  RightPortType=output  PseudoPeriodic=off  Frequencies=[]  NoiseDistribution=none  NoiseParameters=[]  Unit=1  AffineConversion=off  FilteringAndDerivatives=provide  SimscapeFilterOrder=2  InputFilterTimeConstant=0.001  UdotUserProvided=0
  Sum : Sum  Inputs=-+|
  Sum1 : Sum  Inputs=|++
  Unit Delay1 : UnitDelay  SampleTime=Ts  HasFrameUpgradeWarning=on
  h : Gain  Gain=[h_alpha h_beta h_zero]'
  tau : Subsystem  mask{tau=[tau_alpha tau_beta tau_zero]-Ts, Ts=Ts}
      mask prompts: tau "Transport Delay (s)"; Ts "Sample Time (s)"
      mask DialogControl DescGroupVar Prompt: Discrete Transport Delay
      mask DialogControl DescTextVar Prompt: This block implements a discrete transport delay. When the transport delay is not an integer multiple of the sample time, the remainder is averaged.
      mask DialogControl param Prompt: Parameters
      mask Display: disp('Discrete\nTransport\nDelay')
  "tau " : Subsystem  mask{tau=[tau_alpha tau_beta tau_zero]-Ts, Ts=Ts}
      mask prompts: tau "Transport Delay (s)"; Ts "Sample Time (s)"
      mask DialogControl DescGroupVar Prompt: Discrete Transport Delay
      mask DialogControl DescTextVar Prompt: This block implements a discrete transport delay. When the transport delay is not an integer multiple of the sample time, the remainder is averaged.
      mask DialogControl param Prompt: Parameters
      mask Display: disp('Discrete\nTransport\nDelay')
  ~1 : PMIOPort  Side=Right
  send : Outport
  wires:
  Unit Delay1/1 -> Sum1/1
  receive/1 -> Sum1/2
  (1+h)/2/1 -> send/1
  [Vabc] -> Clarke Transform (Transpose)/1
  (1-h)/2/1 -> Unit Delay1/1
  "Clarke Transform "/1 -> [Iabc]
  "tau "/1(y) -> Sum/1
  Sum/1 -> (1-h)/2/1, (1+h)/2/1
  h/1 -> "tau "/1(u)
  tau/1(y) -> (1+h)./(Zlc+R/4)/1
  Sum1/1 -> "Clarke Transform "/1, h/1
  Clarke Transform (Transpose)/1 -> tau/1(u)
  Mux/1 -> [Vabc]
  PS-Simulink Converter2/1 -> Mux/2
  PS-Simulink Converter1/1 -> Mux/1
  PS-Simulink Converter3/1 -> Mux/3
  [Iabc] -> Simulink-PS Converter2/1
  (1+h)./(Zlc+R/4)/1 -> Sum/2
  connections:
  Characteristic Impedance/LConn3 -- Phase Splitter/RConn3
  Characteristic Impedance/LConn2 -- Phase Splitter/RConn2
  Characteristic Impedance/LConn1 -- Phase Splitter/RConn1
  Electrical Reference3/LConn1 -- Characteristic Impedance/RConn2 -- Characteristic Impedance/RConn3 -- Characteristic Impedance/RConn1
  ~1/RConn1 -- Phase Splitter/LConn1 -- Phase Voltage Sensor (Three-Phase)/LConn1 -- Controlled Current Source (Three-Phase)/RConn1
  PS-Simulink Converter3/LConn1 -- PS Three-Element Demux/RConn3
  PS-Simulink Converter2/LConn1 -- PS Three-Element Demux/RConn2
  PS Three-Element Demux/LConn1 -- Phase Voltage Sensor (Three-Phase)/RConn1
  Phase Voltage Sensor (Three-Phase)/RConn2 -- Grounded Neutral (Three-Phase)1/LConn1
  PS-Simulink Converter1/LConn1 -- PS Three-Element Demux/RConn1
  Simulink-PS Converter2/RConn1 -- Controlled Current Source (Three-Phase)/LConn1
  Controlled Current Source (Three-Phase)/LConn2 -- Electrical Reference/LConn1

== system ee_lib_for_power_systems/Network Components/Connector Bergeron Line (single-phase) ==
  note: Reference
      |
      | Dommel, H., “Digital Computer Solution of Electromagnetic Transients in Single and Multiple Networks,” IEEE® Transactions on Power Apparatus and Systems , Vol. PAS-88, No. 4, April, 1969.
  note: Simscape
  note: Simulink
  receive : Inport
  (1+h)./(Zlc+R/4) : Gain  Gain=(1+h)/(Zlc+R/4)
  (1+h)/2 : Gain  Gain=(1+h)/2
  (1-h)/2 : Gain  Gain=(1-h)/2
  "Characteristic Impedance " : fl_lib/Electrical/Electrical Elements/Resistor  R=Zc Ohm  i_specify=off  i_priority=None  i=0 A  i_nominal_specify=off  i_nominal_value=1  i_nominal_unit=A  v_specify=off  v_priority=None  v=0 V  v_nominal_specify=off  v_nominal_value=1  v_nominal_unit=V
  Controlled Current Source1 : fl_lib/Electrical/Electrical Sources/Controlled Current Source
  Electrical Reference : fl_lib/Electrical/Electrical Elements/Electrical Reference
  PS-Simulink Converter1 : nesl_utility/PS-Simulink Converter  PhysicalDomain=network_engine_domain  SubClassName=ps_output  LeftPortType=input  RightPortType=output  PseudoPeriodic=off  Frequencies=[]  VectorFormat=inherit  Unit=inherit  AffineConversion=off
  Simulink-PS Converter2 : nesl_utility/Simulink-PS Converter  PhysicalDomain=network_engine_domain  SubClassName=ps_input  LeftPortType=input  RightPortType=output  PseudoPeriodic=off  Frequencies=[]  NoiseDistribution=none  NoiseParameters=[]  Unit=1  AffineConversion=off  FilteringAndDerivatives=provide  SimscapeFilterOrder=2  InputFilterTimeConstant=0.001  UdotUserProvided=0
  Sum : Sum  Inputs=-+|
  Sum1 : Sum  Inputs=|++
  Unit Delay1 : UnitDelay  SampleTime=Ts  HasFrameUpgradeWarning=on
  Voltage Sensor1 : fl_lib/Electrical/Electrical Sensors/Voltage Sensor
  h : Gain  Gain=h
  tau : Subsystem  mask{tau=tau-Ts, Ts=Ts}
      mask prompts: tau "Transport Delay (s)"; Ts "Sample Time (s)"
      mask DialogControl DescGroupVar Prompt: Discrete Transport Delay
      mask DialogControl DescTextVar Prompt: This block implements a discrete transport delay. When the transport delay is not an integer multiple of the sample time, the remainder is averaged.
      mask DialogControl param Prompt: Parameters
      mask Display: disp('Discrete\nTransport\nDelay')
  "tau " : Subsystem  mask{tau=tau-Ts, Ts=Ts}
      mask prompts: tau "Transport Delay (s)"; Ts "Sample Time (s)"
      mask DialogControl DescGroupVar Prompt: Discrete Transport Delay
      mask DialogControl DescTextVar Prompt: This block implements a discrete transport delay. When the transport delay is not an integer multiple of the sample time, the remainder is averaged.
      mask DialogControl param Prompt: Parameters
      mask Display: disp('Discrete\nTransport\nDelay')
  + : PMIOPort  Side=Left
  send : Outport
  wires:
  Unit Delay1/1 -> Sum1/1
  (1+h)/2/1 -> send/1
  receive/1 -> Sum1/2
  [I] -> Simulink-PS Converter2/1
  PS-Simulink Converter1/1 -> [V]
  [V] -> tau/1(u)
  tau/1(y) -> (1+h)./(Zlc+R/4)/1
  h/1 -> "tau "/1(u)
  Sum/1 -> (1-h)/2/1, (1+h)/2/1
  "tau "/1(y) -> Sum/1
  Sum1/1 -> [I], h/1
  (1+h)./(Zlc+R/4)/1 -> Sum/2
  (1-h)/2/1 -> Unit Delay1/1
  connections:
  Electrical Reference/LConn1 -- "Characteristic Impedance "/RConn1 -- Voltage Sensor1/RConn2 -- Controlled Current Source1/RConn2
  Simulink-PS Converter2/RConn1 -- Controlled Current Source1/RConn1
  Voltage Sensor1/RConn1 -- PS-Simulink Converter1/LConn1
  +/RConn1 -- Voltage Sensor1/LConn1 -- Controlled Current Source1/LConn1 -- "Characteristic Impedance "/LConn1

== system ee_lib_for_power_systems/Network Components/Distributed Parameter Transmission Line (Three-Phase)  ==
  distributed_parameter_line : custom_lib/Distributed Parameter Transmission Line (Three-phase)  port_option=ee.enum.threePhasePort.composite  length=length km  freq=Fnom Hz  R1=R1 Ohm/km  R0=R0 Ohm/km  L1=L1 H/km  L0=L0 H/km  C1=C1 F/km  C0=C0 F/km
      settings: InternalSimscapePortConfiguration="{\"Bottom\":[],\"Left\":[{\"id\":\"N1\",\"label\":\"~1\",\"type\":\"foundation.electrical.three_phase\"}],\"Right\":[{\"id\":\"N2\",\"label\":\"~2\",\"type\":\"foundation.electrical.three_phase\"}],\"Top\":[]}"
  ~1 : PMIOPort  Side=Left
  ~2 : PMIOPort  Port=2  Side=Right
  connections:
  distributed_parameter_line/LConn1 -- ~1/RConn1
  distributed_parameter_line/RConn1 -- ~2/RConn1

== system ee_lib_for_power_systems/Network Components/Dynamic Load ==
  PQ : Inport
  Demux : Demux  Outputs=2
  Simulink-PS Converter : nesl_utility/Simulink-PS Converter  PhysicalDomain=network_engine_domain  SubClassName=ps_input  LeftPortType=input  RightPortType=output  PseudoPeriodic=off  Frequencies=[]  NoiseDistribution=none  NoiseParameters=[]  Unit=1  AffineConversion=off  FilteringAndDerivatives=zero  SimscapeFilterOrder=1  InputFilterTimeConstant=0.001  UdotUserProvided=0
  Simulink-PS Converter1 : nesl_utility/Simulink-PS Converter  PhysicalDomain=network_engine_domain  SubClassName=ps_input  LeftPortType=input  RightPortType=output  PseudoPeriodic=off  Frequencies=[]  NoiseDistribution=none  NoiseParameters=[]  Unit=1  AffineConversion=off  FilteringAndDerivatives=zero  SimscapeFilterOrder=1  InputFilterTimeConstant=0.001  UdotUserProvided=0
  pq_load : ee_lib/Passive/Dynamic Load (Three-Phase)  port_option=ee.enum.threePhasePort.composite  FRated=Fnom Hz  Vline_rms_min=1 V  tau=0.01 s  Vline_rms_ini=Vnom V  Vang_ini=0 deg  gmin=0 S
      settings: InternalSimscapePortConfiguration="{\"Bottom\":[],\"Left\":[{\"id\":\"N\",\"label\":\"~\",\"type\":\"foundation.electrical.three_phase\"}],\"Right\":[],\"Top\":[{\"id\":\"P\",\"label\":\"P\",\"type\":\"input\"},{\"id\":\"Q\",\"label\":\"Q\",\"type\":\"input\"}]}"
  ~ : PMIOPort  Side=Left
  wires:
  Demux/2 -> Simulink-PS Converter1/1
  PQ/1 -> Demux/1
  Demux/1 -> Simulink-PS Converter/1
  connections:
  Simulink-PS Converter/RConn1 -- pq_load/LConn2
  pq_load/LConn1 -- ~/RConn1
  Simulink-PS Converter1/RConn1 -- pq_load/LConn3

== system ee_lib_for_power_systems/Network Components/Linear Transformer (PU) ==
  Ideal Transformer : fl_lib/Electrical/Electrical Elements/Ideal Transformer  n=V1/V2  i1_specify=off  i1_priority=None  i1=0 A  i1_nominal_specify=off  i1_nominal_value=1  i1_nominal_unit=A  v1_specify=off  v1_priority=None  v1=0 V  v1_nominal_specify=off  v1_nominal_value=1  v1_nominal_unit=V  i2_specify=off  i2_priority=None  i2=0 A  i2_nominal_specify=off  i2_nominal_value=1  i2_nominal_unit=A  v2_specify=off  v2_priority=None  v2=0 V  v2_nominal_specify=off  v2_nominal_value=1  v2_nominal_unit=V
  L1 : fl_lib/Electrical/Electrical Elements/Inductor  l=L1_si H  r=0 Ohm  g=0 1/Ohm  i_specify=off  i_priority=None  i=0 A  i_nominal_specify=off  i_nominal_value=1  i_nominal_unit=A  v_specify=off  v_priority=None  v=0 V  v_nominal_specify=off  v_nominal_value=1  v_nominal_unit=V  i_L_specify=off  i_L_priority=High  i_L=0 A  i_L_nominal_specify=off  i_L_nominal_value=1  i_L_nominal_unit=A
  L2 : fl_lib/Electrical/Electrical Elements/Inductor  l=L2_si H  r=0 Ohm  g=0 1/Ohm  i_specify=off  i_priority=None  i=0 A  i_nominal_specify=off  i_nominal_value=1  i_nominal_unit=A  v_specify=off  v_priority=None  v=0 V  v_nominal_specify=off  v_nominal_value=1  v_nominal_unit=V  i_L_specify=off  i_L_priority=High  i_L=0 A  i_L_nominal_specify=off  i_L_nominal_value=1  i_L_nominal_unit=A
  Lm : fl_lib/Electrical/Electrical Elements/Inductor  l=Lm_si H  r=0 Ohm  g=0 1/Ohm  i_specify=off  i_priority=None  i=0 A  i_nominal_specify=off  i_nominal_value=1  i_nominal_unit=A  v_specify=off  v_priority=None  v=0 V  v_nominal_specify=off  v_nominal_value=1  v_nominal_unit=V  i_L_specify=off  i_L_priority=High  i_L=0 A  i_L_nominal_specify=off  i_L_nominal_value=1  i_L_nominal_unit=A
  R1 : fl_lib/Electrical/Electrical Elements/Resistor  R=R1_si Ohm  i_specify=off  i_priority=None  i=0 A  i_nominal_specify=off  i_nominal_value=1  i_nominal_unit=A  v_specify=off  v_priority=None  v=0 V  v_nominal_specify=off  v_nominal_value=1  v_nominal_unit=V
  R2 : fl_lib/Electrical/Electrical Elements/Resistor  R=R2_si Ohm  i_specify=off  i_priority=None  i=0 A  i_nominal_specify=off  i_nominal_value=1  i_nominal_unit=A  v_specify=off  v_priority=None  v=0 V  v_nominal_specify=off  v_nominal_value=1  v_nominal_unit=V
  Rm : fl_lib/Electrical/Electrical Elements/Resistor  R=Rm_si Ohm  i_specify=off  i_priority=None  i=0 A  i_nominal_specify=off  i_nominal_value=1  i_nominal_unit=A  v_specify=off  v_priority=None  v=0 V  v_nominal_specify=off  v_nominal_value=1  v_nominal_unit=V
  1+ : PMIOPort  Side=Left
  2+ : PMIOPort  Port=2  Side=Right
  1- : PMIOPort  Port=3  Side=Left
  2- : PMIOPort  Port=4  Side=Right
  connections:
  1-/RConn1 -- Rm/RConn1 -- Ideal Transformer/LConn2 -- Lm/RConn1
  Ideal Transformer/LConn1 -- Rm/LConn1 -- L1/RConn1 -- Lm/LConn1
  R1/LConn1 -- 1+/RConn1
  L2/RConn1 -- 2+/RConn1
  L2/LConn1 -- R2/RConn1
  L1/LConn1 -- R1/RConn1
  Ideal Transformer/RConn2 -- 2-/RConn1
  Ideal Transformer/RConn1 -- R2/LConn1

== system ee_lib_for_power_systems/Network Components/Mutual RL Pos-Zero (PU) ==
  Coupled : ee_lib/Passive/Lines/Coupled Lines (Three-Phase)  port_option=ee.enum.threePhasePort.composite  prm=1  La=1e-3 H  Lb=1e-3 H  Lc=1e-3 H  L=Ls_si H  Lmab=3e-4 H  Lmac=3e-4 H  Lmbc=3e-4 H  Lm=Lm_si H  Ra=0.001 Ohm  Rb=0.001 Ohm  Rc=0.001 Ohm  R=Rs_si Ohm  Rmab=0 Ohm  Rmac=0 Ohm  Rmbc=0 Ohm  Rm=Rm_si Ohm  Ga=1e-9 1/Ohm  Gb=1e-9 1/Ohm  Gc=1e-9 1/Ohm  G=0 1/Ohm  I_L_specify=off  I_L_priority=High  I_L=[0, 0, 0] A  I_L_nominal_specify=off  I_L_nominal_value=1  I_L_nominal_unit=A  i_La_specify=off  i_La_priority=High  i_La=0 A  i_La_nominal_specify=off  i_La_nominal_value=1  i_La_nominal_unit=A  i_Lb_specify=off  i_Lb_priority=High  i_Lb=0 A  i_Lb_nominal_specify=off  i_Lb_nominal_value=1  i_Lb_nominal_unit=A  i_Lc_specify=off  i_Lc_priority=High  i_Lc=0 A  i_Lc_nominal_specify=off  i_Lc_nominal_value=1  i_Lc_nominal_unit=A
      settings: InternalSimscapePortConfiguration="{\"Bottom\":[],\"Left\":[{\"id\":\"N1\",\"label\":\"~1\",\"type\":\"foundation.electrical.three_phase\"}],\"Right\":[{\"id\":\"N2\",\"label\":\"~2\",\"type\":\"foundation.electrical.three_phase\"}],\"Top\":[]}"
  ~1 : PMIOPort  Side=Left
  ~2 : PMIOPort  Port=2  Side=Right
  connections:
  Coupled/LConn1 -- ~1/RConn1
  Coupled/RConn1 -- ~2/RConn1

== system ee_lib_for_power_systems/Network Components/Mutual RL Pos-Zero (SI) ==
  Coupled : ee_lib/Passive/Lines/Coupled Lines (Three-Phase)  port_option=ee.enum.threePhasePort.composite  prm=1  La=1e-3 H  Lb=1e-3 H  Lc=1e-3 H  L=Ls_si H  Lmab=3e-4 H  Lmac=3e-4 H  Lmbc=3e-4 H  Lm=Lm_si H  Ra=0.001 Ohm  Rb=0.001 Ohm  Rc=0.001 Ohm  R=Rs_si Ohm  Rmab=0 Ohm  Rmac=0 Ohm  Rmbc=0 Ohm  Rm=Rm_si Ohm  Ga=1e-9 1/Ohm  Gb=1e-9 1/Ohm  Gc=1e-9 1/Ohm  G=0 1/Ohm  I_L_specify=off  I_L_priority=High  I_L=[0, 0, 0] A  I_L_nominal_specify=off  I_L_nominal_value=1  I_L_nominal_unit=A  i_La_specify=off  i_La_priority=High  i_La=0 A  i_La_nominal_specify=off  i_La_nominal_value=1  i_La_nominal_unit=A  i_Lb_specify=off  i_Lb_priority=High  i_Lb=0 A  i_Lb_nominal_specify=off  i_Lb_nominal_value=1  i_Lb_nominal_unit=A  i_Lc_specify=off  i_Lc_priority=High  i_Lc=0 A  i_Lc_nominal_specify=off  i_Lc_nominal_value=1  i_Lc_nominal_unit=A
      settings: InternalSimscapePortConfiguration="{\"Bottom\":[],\"Left\":[{\"id\":\"N1\",\"label\":\"~1\",\"type\":\"foundation.electrical.three_phase\"}],\"Right\":[{\"id\":\"N2\",\"label\":\"~2\",\"type\":\"foundation.electrical.three_phase\"}],\"Top\":[]}"
  ~1 : PMIOPort  Side=Left
  ~2 : PMIOPort  Port=2  Side=Right
  connections:
  Coupled/RConn1 -- ~2/RConn1
  Coupled/LConn1 -- ~1/RConn1

== system ee_lib_for_power_systems/Network Components/Parallel Z - PQ ==
  Capacitor : fl_lib/Electrical/Electrical Elements/Capacitor  Commented=on  c=C F  r=0 Ohm  g=0 1/Ohm  i_specify=off  i_priority=None  i=0 A  i_nominal_specify=off  i_nominal_value=1  i_nominal_unit=A  v_specify=off  v_priority=None  v=0 V  v_nominal_specify=off  v_nominal_value=1  v_nominal_unit=V  vc_specify=off  vc_priority=High  vc=0 V  vc_nominal_specify=off  vc_nominal_value=1  vc_nominal_unit=V
  Inductor : fl_lib/Electrical/Electrical Elements/Inductor  l=L H  r=0 Ohm  g=0 1/Ohm  i_specify=off  i_priority=None  i=0 A  i_nominal_specify=off  i_nominal_value=1  i_nominal_unit=A  v_specify=off  v_priority=None  v=0 V  v_nominal_specify=off  v_nominal_value=1  v_nominal_unit=V  i_L_specify=off  i_L_priority=High  i_L=0 A  i_L_nominal_specify=off  i_L_nominal_value=1  i_L_nominal_unit=A
  Resistor : fl_lib/Electrical/Electrical Elements/Resistor  R=R Ohm  i_specify=off  i_priority=None  i=0 A  i_nominal_specify=off  i_nominal_value=1  i_nominal_unit=A  v_specify=off  v_priority=None  v=0 V  v_nominal_specify=off  v_nominal_value=1  v_nominal_unit=V
  + : PMIOPort  Side=Left
  - : PMIOPort  Port=2  Side=Right
  connections:
  +/RConn1 -- Resistor/LConn1 -- Capacitor/LConn1 -- Inductor/LConn1
  Resistor/RConn1 -- -/RConn1 -- Capacitor/RConn1 -- Inductor/RConn1
